simulink model "Complete_Model"  (R2023a)
settings: Solver=VariableStepAuto  StartTime=0.0  StopTime=22  MinStep=5e-5  RelTol=1e-3
values from neighbouring files (symbols this model uses):
  Cbus = 80e-3*n_wt_agg*u^2    (= 1.33333333333)    [param.m]
  D = 1e-3    (= 0.001)    [param.m]
  f = 50    [param.m]
  Fs = Vs * sqrt(2/3)/(2*pi*f)    [param.m]
  fsw = 4e3    (= 4000)    [param.m]
  Idr_rated = sqrt(2)*Is    [param.m]
  J = 127*n_wt_agg    (= 19050)    [param.m]
  ki_id = (wni^2)*Lr*sigma    (= 3.27733001332)    [param.m]
  ki_idg = (wnig^2)*Lg    (= 0.263189450696)    [param.m]
  ki_iq = ki_id    (= 3.27733001332)    [param.m]
  ki_iqg = ki_idg    (= 0.263189450696)    [param.m]
  ki_v = -300000*n_wt_agg    (= -45000000)    [param.m]
  Kopt = ((0.5*rho*pi*(Radius^5)*Cp_max)/(lambda_opt^3))    (= 296454.198186)    [param.m]
  kp_id = (2*wni*sigma*Lr) - Rr    (= 0.00384733333333)    [param.m]
  kp_idg = ((2*wnig*Lg)-Rg)    (= 0.00167538274858)    [param.m]
  kp_iq = kp_id    (= 0.00384733333333)    [param.m]
  kp_iqg = kp_idg    (= 0.00167538274858)    [param.m]
  kp_v = -1000*n_wt_agg    (= -150000)    [param.m]
  Kpg = 1/(1.5*Vr*sqrt(2/3))    [param.m]
  Kqg = -Kpg    [param.m]
  lambda_opt = 7.2    [param.m]
  Lg = 400e-6 /n_wt_agg    (= 2.66666666667e-06)    [param.m]
  Lm = 2.5e-3 /n_wt_agg    (= 1.66666666667e-05)    [param.m]
  Lr = Lm + Lsi    (= 1.72466666667e-05)    [param.m]
  Ls = Lm + Lsi    (= 1.72466666667e-05)    [param.m]
  Lsi = 0.087e-3 /n_wt_agg    (= 5.8e-07)    [param.m]
  N = 100    [param.m]
  n_wt_agg = 150    [param.m]
  p = 2    [param.m]
  Ps = 2e6*n_wt_agg    (= 300000000)    [param.m]
  Radius = 42    [param.m]
  Rg = 20e-6 /n_wt_agg    (= 1.33333333333e-07)    [param.m]
  rho = 1.225    [param.m]
  Rr = 2.9e-3 /n_wt_agg    (= 1.93333333333e-05)    [param.m]
  Rs = 2.6e-3 /n_wt_agg    (= 1.73333333333e-05)    [param.m]
  sigma = 1 - Lm^2 / (Ls*Lr)    (= 0.0661284179513)    [param.m]
  smax = 1/3    (= 0.333333333333)    [param.m]
  tab_lambda = [0.1:0.01:11.8]    [param.m]
  Vbus = 1150/u    (= 3450)    [param.m]
  Vs = 690    [param.m]
undefined symbols (not defined in the file or in its folder): Cp, D1, Delta, DiscIntNeverNeededParam, magnitude
libraries: spsSecondOrderFilterLib x133, spsThreePhaseVIMeasurementLib x58, spsPower3phInstantaneousLib x52, simulink x41, spsThreePhaseTransformerTwoWindingsLib x27, spsUniversalBridgeLib x22, spsPWMGenerator2LevelLib x22, spsSeriesRLCBranchLib x19, spsFirstOrderFilterLib x18, spsThreePhasePISectionLineLib x17, spsGroundLib x17, spsThreePhaseSeriesRLCBranchLib x17
(part 6 of 15)

== system Complete_Model/HVDC Link/Wind farm side control ==
  note: Active Power Control
  note: Reactive Power Control
  P_windfarm : Inport
  Pref_windfarm : Inport  Port=2
  Q_windfarm : Inport  Port=3
  Qref_windfarm : Inport  Port=4
  Clarke - Park Transform : eeTransforms/Park Transform  AxisAlignment=D-axis  PowerInvariant=off
      settings: LibrarySourceBlock=|
      | ee_sl_lib/Mathematical
      | Transforms/Park Transform
  Clarke - Park Transform1 : eeTransforms/Park Transform  AxisAlignment=D-axis  PowerInvariant=off
      settings: LibrarySourceBlock=|
      | ee_sl_lib/Mathematical
      | Transforms/Park Transform
  Constant : Constant  Value=0
  Constant1 : Constant  Value=0
  Demux{1..7} (7 alike) : Demux  Outputs=2
      Demux2 settings: DisplayOption=none
      Demux3 settings: DisplayOption=none
  Divide : Product  Inputs=*/
  Divide2 : Product  Inputs=*/
  First-Order Filter{1,3..11} (10 alike) : spsFirstOrderFilterLib/First-Order Filter  FilterType=Lowpass  Tc=0.015915  Ts=7.4074e-05  Initialize=on  Vac_Init=[0, 0, 50]  Vdc_Init=1.2247  PlotResponse=off  FreqRange="[0,  200,  1]"
      settings: AttributesFormatString=Tc:%<Tc>\nIC:%<Vdc_Init>
  First-Order Filter13 : spsFirstOrderFilterLib/First-Order Filter  FilterType=Lowpass  Tc=0.015915*2  Ts=7.4074e-05  Initialize=on  Vac_Init=[0, 0, 50]  Vdc_Init=1.2247  PlotResponse=off  FreqRange="[0,  200,  1]"
      settings: AttributesFormatString=Tc:%<Tc>\nIC:%<Vdc_Init>
  First-Order Filter2 : spsFirstOrderFilterLib/First-Order Filter  FilterType=Lowpass  Tc=0.015915*2  Ts=7.4074e-05  Initialize=on  Vac_Init=[0, 0, 50]  Vdc_Init=1.2247  PlotResponse=off  FreqRange="[0,  200,  1]"
      settings: AttributesFormatString=Tc:%<Tc>\nIC:%<Vdc_Init>
  Gain : Gain  Gain=0.1947+0.178
  Gain1 : Gain  Gain=0.1947+0.178
  Gain3 : Gain  Gain=-1
  Gain5 : Gain  Gain=-1
  I_max : Constant  Value=1.1
  Inverse Clarke - Park Transform : eeTransforms/Inverse Park Transform  AxisAlignment=D-axis  PowerInvariant=off
      settings: LibrarySourceBlock=|
      | ee_sl_lib/Mathematical
      | Transforms/Inverse
      | Park Transform
  Math Function3 : Math  Operator=magnitude^2  SignedPower=on
  Math Function4 : Math  Operator=magnitude^2  SignedPower=on
  Math Function5 : Math  Operator=sqrt  SignedPower=on
  Mux : Mux  Inputs=3
  Mux1 : Mux  Inputs=2
  PID Controller2 : slpidlib/PID Controller  Controller=PI  Form=Parallel  TimeDomain=Continuous-time  UseExternalTs=off  SampleTime=-1  IntegratorMethod=Forward Euler  FilterMethod=Forward Euler  ControllerParametersSource=internal  P=2  I=4  UseKiTs=off  D=0  N=100  UseFilter=on  TunerSelectOption=Transfer Function Based (PID Tuner App)  InitialConditionSource=internal  InitialConditionForIntegrator=0  InitialConditionForFilter=0  DifferentiatorICPrevScaledInput=0  ExternalReset=none  TrackingMode=off  Kt=1  LimitOutput=on  SatLimitsSource=internal  UpperSaturationLimit=1.1  LowerSaturationLimit=-1.1  LinearizeAsGain=on  AntiWindupMode=clamping  Kb=1  LimitIntegrator=on  UpperIntegratorSaturationLimit=20  LowerIntegratorSaturationLimit=-20  POutMin=[]  POutMax=[]  IOutMin=[]  IOutMax=[]  DOutMin=[]  DOutMax=[]  NOutMin=[]  NOutMax=[]  SumOutMin=[]  SumOutMax=[]  SaturationOutMin=[]  SaturationOutMax=[]  PParamMin=[]  PParamMax=[]  IParamMin=[]  IParamMax=[]  DParamMin=[]  DParamMax=[]  NParamMin=[]  NParamMax=[]  KbParamMin=[]  KbParamMax=[]  KtParamMin=[]  KtParamMax=[]  KbOutMin=[]  KbOutMax=[]  KtOutMin=[]  KtOutMax=[]  IntegratorOutMin=[]  IntegratorOutMax=[]  FilterOutMin=[]  FilterOutMax=[]  SumI1OutMin=[]  SumI1OutMax=[]  SumI2OutMin=[]  SumI2OutMax=[]  SumI3OutMin=[]  SumI3OutMax=[]  SumI4OutMin=[]  SumI4OutMax=[]  SumDOutMin=[]  SumDOutMax=[]  DifferentiatorOutMin=[]  DifferentiatorOutMax=[]  FilterDiffOutCoefMin=[]  FilterDiffOutCoefMax=[]  ReciprocalOutMin=[]  ReciprocalOutMax=[]  SumDenOutMin=[]  SumDenOutMax=[]  SumNumOutMin=[]  SumNumOutMax=[]  DivideOutMin=[]  DivideOutMax=[]  UdiffTsProdOutMin=[]  UdiffTsProdOutMax=[]  NTsProdOutMin=[]  NTsProdOutMax=[]  UintegralTsProdOutMin=[]  UintegralTsProdOutMax=[]  UngainTsProdOutMin=[]  UngainTsProdOutMax=[]  ClampingZeroOutDataTypeStr=Inherit: Inherit via back propagation  ClampingZeroOutMin=[]  ClampingZeroOutMax=[]  FilterDenConstantOutDataTypeStr=Inherit: Inherit via back propagation  FilterDenConstantOutMin=[]  FilterDenConstantOutMax=[]  PCopyOutDataTypeStr=Inherit: Inherit via back propagation  PCopyOutMin=[]  PCopyOutMax=[]  NCopyOutDataTypeStr=Inherit: Inherit via back propagation  NCopyOutMin=[]  NCopyOutMax=[]  IntegratorContinuousStateAttributes=''  IntegratorStateMustResolveToSignalObject=off  IntegratorRTWStateStorageClass=Auto  FilterContinuousStateAttributes=''  FilterStateMustResolveToSignalObject=off  FilterRTWStateStorageClass=Auto  ParallelPVariant=InternalParameters  IdealPVariant=Disabled  IVariant=InternalParameters  DVariant=Disabled  IntegratorVariant=Continuous  SatVariant=Enabled  AWVariant=ContClampingParallel  PCopyVariant=Disabled  TRVariant=Disabled  FdbkBlocksVariant=Forward  IdealPFdbkVariant=Disabled  SatFdbkVariant=Disabled  DerivativeFilterVariant=Disabled  NVariant=Disabled  NCopyVariant=Disabled_SignalSpec  FilterICVariant=Disabled  IntegratorICVariant=InternalICs  ExternalResetVariant=Disabled  TRSumVariant=Passthrough  SumFdbkVariant=Disabled  SumVariant=Sum_PI  TsampFilterVariant=InternalTs  TsampNgainVariant=Passthrough  TsampIntegralVariant=Disabled_SignalSpec  ExternalSaturationOutDataTypeStr=Inherit: Same as second input
  PID Controller5 : slpidlib/PID Controller  Controller=PI  Form=Parallel  TimeDomain=Continuous-time  UseExternalTs=off  SampleTime=-1  IntegratorMethod=Forward Euler  FilterMethod=Forward Euler  ControllerParametersSource=internal  P=2  I=10  UseKiTs=off  D=0  N=100  UseFilter=on  TunerSelectOption=Transfer Function Based (PID Tuner App)  InitialConditionSource=internal  InitialConditionForIntegrator=0  InitialConditionForFilter=0  DifferentiatorICPrevScaledInput=0  ExternalReset=none  TrackingMode=off  Kt=1  LimitOutput=on  SatLimitsSource=internal  UpperSaturationLimit=1.1  LowerSaturationLimit=-1.1  LinearizeAsGain=on  AntiWindupMode=clamping  Kb=1  LimitIntegrator=on  UpperIntegratorSaturationLimit=20  LowerIntegratorSaturationLimit=-20  POutMin=[]  POutMax=[]  IOutMin=[]  IOutMax=[]  DOutMin=[]  DOutMax=[]  NOutMin=[]  NOutMax=[]  SumOutMin=[]  SumOutMax=[]  SaturationOutMin=[]  SaturationOutMax=[]  PParamMin=[]  PParamMax=[]  IParamMin=[]  IParamMax=[]  DParamMin=[]  DParamMax=[]  NParamMin=[]  NParamMax=[]  KbParamMin=[]  KbParamMax=[]  KtParamMin=[]  KtParamMax=[]  KbOutMin=[]  KbOutMax=[]  KtOutMin=[]  KtOutMax=[]  IntegratorOutMin=[]  IntegratorOutMax=[]  FilterOutMin=[]  FilterOutMax=[]  SumI1OutMin=[]  SumI1OutMax=[]  SumI2OutMin=[]  SumI2OutMax=[]  SumI3OutMin=[]  SumI3OutMax=[]  SumI4OutMin=[]  SumI4OutMax=[]  SumDOutMin=[]  SumDOutMax=[]  DifferentiatorOutMin=[]  DifferentiatorOutMax=[]  FilterDiffOutCoefMin=[]  FilterDiffOutCoefMax=[]  ReciprocalOutMin=[]  ReciprocalOutMax=[]  SumDenOutMin=[]  SumDenOutMax=[]  SumNumOutMin=[]  SumNumOutMax=[]  DivideOutMin=[]  DivideOutMax=[]  UdiffTsProdOutMin=[]  UdiffTsProdOutMax=[]  NTsProdOutMin=[]  NTsProdOutMax=[]  UintegralTsProdOutMin=[]  UintegralTsProdOutMax=[]  UngainTsProdOutMin=[]  UngainTsProdOutMax=[]  ClampingZeroOutDataTypeStr=Inherit: Inherit via back propagation  ClampingZeroOutMin=[]  ClampingZeroOutMax=[]  FilterDenConstantOutDataTypeStr=Inherit: Inherit via back propagation  FilterDenConstantOutMin=[]  FilterDenConstantOutMax=[]  PCopyOutDataTypeStr=Inherit: Inherit via back propagation  PCopyOutMin=[]  PCopyOutMax=[]  NCopyOutDataTypeStr=Inherit: Inherit via back propagation  NCopyOutMin=[]  NCopyOutMax=[]  IntegratorContinuousStateAttributes=''  IntegratorStateMustResolveToSignalObject=off  IntegratorRTWStateStorageClass=Auto  FilterContinuousStateAttributes=''  FilterStateMustResolveToSignalObject=off  FilterRTWStateStorageClass=Auto  ParallelPVariant=InternalParameters  IdealPVariant=Disabled  IVariant=InternalParameters  DVariant=Disabled  IntegratorVariant=Continuous  SatVariant=Enabled  AWVariant=ContClampingParallel  PCopyVariant=Disabled  TRVariant=Disabled  FdbkBlocksVariant=Forward  IdealPFdbkVariant=Disabled  SatFdbkVariant=Disabled  DerivativeFilterVariant=Disabled  NVariant=Disabled  NCopyVariant=Disabled_SignalSpec  FilterICVariant=Disabled  IntegratorICVariant=InternalICs  ExternalResetVariant=Disabled  TRSumVariant=Passthrough  SumFdbkVariant=Disabled  SumVariant=Sum_PI  TsampFilterVariant=InternalTs  TsampNgainVariant=Passthrough  TsampIntegralVariant=Disabled_SignalSpec  ExternalSaturationOutDataTypeStr=Inherit: Same as second input
  PI_regulator 1 : Subsystem  mask(PI regulator with anti-windup - type 2){max_i=2, min_i=-2, Ki=6, i_ic=0, Kp=0.6, Kf=1.0, max_out=2, min_out=-2, Ts=7.4074e-05}
      mask prompts: max_i "Integrator maximum  limit (pu):"; min_i "Integrator minimum  limit (pu):"; Ki "Integrator gain (pu/s):"; i_ic "Integrator initial condition (pu):"; Kp "Proportional gain:"; Kf "Feadback gain :"; max_out "Output maximum limit (pu):"; min_out "Output minimum limit (pu):"; Ts "Sample time: (s)"
      mask help:
      | The error signal (input 1 - input 2) is integrated with dynamic limits (Integrator min and max limits).
      | A back-calculation anti-windup method discharges the integrator on block saturation (output min and max limits) using a feedback loop having a gain coefficient.
      mask Display: disp('PI \n(Type 2)')
  PI_regulator 2 : Subsystem  mask(PI regulator with anti-windup - type 2){max_i=2, min_i=-2, Ki=6, i_ic=0, Kp=0.6, Kf=1.0, max_out=2, min_out=-2, Ts=7.4074e-05}
      mask prompts: max_i "Integrator maximum  limit (pu):"; min_i "Integrator minimum  limit (pu):"; Ki "Integrator gain (pu/s):"; i_ic "Integrator initial condition (pu):"; Kp "Proportional gain:"; Kf "Feadback gain :"; max_out "Output maximum limit (pu):"; min_out "Output minimum limit (pu):"; Ts "Sample time: (s)"
      mask help:
      | The error signal (input 1 - input 2) is integrated with dynamic limits (Integrator min and max limits).
      | A back-calculation anti-windup method discharges the integrator on block saturation (output min and max limits) using a feedback loop having a gain coefficient.
      mask Display: disp('PI \n(Type 2)')
  Park Transform3 : eeTransforms/Park Transform  AxisAlignment=D-axis  PowerInvariant=on
      settings: LibrarySourceBlock=|
      | ee_sl_lib/Mathematical
      | Transforms/Park Transform
  Saturation : Saturate  UpperLimit=1.1  LowerLimit=-1.1
  Saturation Dynamic : simulink/Discontinuities/Saturation Dynamic  OutMin=[]  OutMax=[]  OutDataTypeStr=Inherit: Same as second input  OutDataType=fixdt(1, 16)  OutScaling=2^-10  DoSatur=off
  Saturation3 : Saturate  UpperLimit=1.1  LowerLimit=-1.1
  Scope12 : Scope
  Scope3 : Scope  NumInputPorts=2
  Selector1 : Selector  InputPortWidth=3  IndexOptions=Index vector (dialog)  Indices=[1 2]  OutputSizes=1
  Selector2 : Selector  InputPortWidth=3  IndexOptions=Index vector (dialog)  Indices=[1 2]  OutputSizes=1
  Selector3 : Selector  InputPortWidth=3  IndexOptions=Index vector (dialog)  Indices=[1 2]  OutputSizes=1
  Simple PLL : Trigonometry  Operator=atan2
  Simple PLL1 : Trigonometry  Operator=atan2
  Step : Step  Time=0.3  Before=1  After=-1  SampleTime=0
  Sum1 : Sum  Inputs=+|-|+  InputSameDT=on  SaturateOnIntegerOverflow=on  OutDataTypeStr=Inherit: Same as first input
  Sum2 : Sum  Inputs=-+  SaturateOnIntegerOverflow=on
  Sum3 : Sum  Inputs=--+  InputSameDT=on  SaturateOnIntegerOverflow=on  OutDataTypeStr=Inherit: Same as first input
  Sum4 : Sum  Inputs=+-|
  Sum5 : Sum  Inputs=++|
  Sum7 : Sum  Inputs=+-|
  Sum9 : Sum  Inputs=++|
  Switch : Switch  Criteria=u2 > Threshold  SaturateOnIntegerOverflow=off  InputSameDT=off
  To File : ToFile  Filename=Iabc_windfarm_hvdc  SaveFormat=Timeseries
  To Workspace : ToWorkspace  VariableName=Ewind_d  SaveFormat=Timeseries  SampleTime=-1
  To Workspace1 : ToWorkspace  VariableName=Ewind_q  SaveFormat=Timeseries  SampleTime=-1
  To Workspace2 : ToWorkspace  VariableName=Iwind_q  SaveFormat=Timeseries  SampleTime=-1
  To Workspace3 : ToWorkspace  VariableName=Iwind_d  SaveFormat=Timeseries  SampleTime=-1
  To Workspace4 : ToWorkspace  VariableName=Iwind_ref_d  SaveFormat=Timeseries  SampleTime=-1
  To Workspace5 : ToWorkspace  VariableName=Iwind_ref_q  SaveFormat=Timeseries  SampleTime=-1
  To Workspace6 : ToWorkspace  VariableName=Ewind_ref_d  SaveFormat=Timeseries  SampleTime=-1
  To Workspace7 : ToWorkspace  VariableName=Ewind_ref_q  SaveFormat=Timeseries  SampleTime=-1
  To Workspace8 : ToWorkspace  VariableName=Uconv_wind_d  SaveFormat=Timeseries  SampleTime=-1
  To Workspace9 : ToWorkspace  VariableName=Uconv_wind_q  SaveFormat=Timeseries  SampleTime=-1
  ToFile_Ewind_d : ToFile  Filename=Ewind_d.mat  SaveFormat=Timeseries
  ToFile_Ewind_q : ToFile  Filename=Ewind_q.mat  SaveFormat=Timeseries
  ToFile_Ewind_ref_d : ToFile  Filename=Ewind_ref_d.mat  SaveFormat=Timeseries
  ToFile_Ewind_ref_q : ToFile  Filename=Ewind_ref_q.mat  SaveFormat=Timeseries
  ToFile_Iwind_d : ToFile  Filename=Iwind_d.mat  SaveFormat=Timeseries
  ToFile_Iwind_q : ToFile  Filename=Iwind_q.mat  SaveFormat=Timeseries
  ToFile_Iwind_ref_d : ToFile  Filename=Iwind_ref_d.mat  SaveFormat=Timeseries
  ToFile_Iwind_ref_q : ToFile  Filename=Iwind_ref_q.mat  SaveFormat=Timeseries
  ToFile_Uconv_wind_d : ToFile  Filename=Uconv_wind_d.mat  SaveFormat=Timeseries
  ToFile_Uconv_wind_q : ToFile  Filename=Uconv_wind_q.mat  SaveFormat=Timeseries
  abc->alpha/beta Clarke : Subsystem
  abc->alpha/beta1 : Subsystem
  Vref_abc : Outport
  tag scope: Ewindfarm_dq=global, Idmes_hvdc_wf=global, Idref_hvdc_wf=global, Iqmes_hvdc_wf=global, Iqref_hvdc_wf=global, Iwindfarm_dq=global, Vdref_hvdc_wf=global, Vqref_hvdc_wf=global, theta_atan_windfarm=global
  wires:
  Constant/1 -> Mux/3
  Inverse Clarke - Park Transform/1 -> Vref_abc/1
  [Ewindfarm_d] -> Sum1/1
  Mux/1 -> Inverse Clarke - Park Transform/1
  Gain1/1 -> Sum3/2
  PI_regulator 2/1(Out) -> Sum3/1
  Demux3/1 -> Gain1/1, PI_regulator 1/1(Ord)  "I_ref_d"
  [Ewindfarm_q] -> Sum3/3
  Gain/1 -> Sum1/3
  PI_regulator 1/1(Out) -> Sum1/2
  Demux2/2 -> PI_regulator 2/2(Meas)  "Iv_q"
  Demux2/1 -> PI_regulator 1/2(Meas)  "Iv_d"
  Demux3/2 -> PI_regulator 2/1(Ord), Gain/1  "I_ref_q"
  Saturation/1 -> Math Function4/1, [Idref_hvdc_wf], Mux1/1, First-Order Filter7/1
  Math Function4/1 -> Sum2/1
  Sum2/1 -> Math Function5/1
  Math Function5/1 -> Gain3/1, Saturation Dynamic/1  "iqmax"
  Math Function3/1 -> Sum2/2
  Mux1/1 -> Demux3/1  "Idq_ref"
  I_max/1 -> Math Function3/1
  P_windfarm/1 -> Sum7/2
  Pref_windfarm/1 -> Divide/1, Sum7/1
  [Ewindfarm_d] -> Divide/2
  Divide/1 -> First-Order Filter2/1
  Saturation Dynamic/1 -> Mux1/2, First-Order Filter6/1, [Iqref_hvdc_wf]
  Switch/1 -> Saturation Dynamic/2
  Gain5/1 -> Switch/3
  Constant1/1 -> Switch/1
  Q_windfarm/1 -> Sum4/2
  Qref_windfarm/1 -> Divide2/1, Sum4/1
  Step/1 -> Switch/2
  Sum4/1 -> PID Controller2/1
  Gain3/1 -> Saturation Dynamic/3
  Demux5/2 -> [Ewindfarm_q], First-Order Filter4/1
  Demux5/1 -> [Ewindfarm_d], First-Order Filter5/1
  Demux4/1 -> Simple PLL/2
  Clarke - Park Transform/1 -> Selector2/1
  [Eabc_windfarm] -> Clarke - Park Transform/1, abc->alpha/beta Clarke/1(abc)
  Demux4/2 -> Simple PLL/1
  Simple PLL/1 -> [theta_atan_windfarm], Clarke - Park Transform/2  "theta_g"
  abc->alpha/beta Clarke/1(alpha/beta) -> Demux4/1
  [Iabc_windfarm] -> To File/1, Clarke - Park Transform1/1
  Selector2/1 -> [Ewindfarm_dq], Demux5/1  "E_dq"
  Clarke - Park Transform1/1 -> Selector1/1
  Demux1/2 -> First-Order Filter8/1, [Iqmes_hvdc_wf]
  Selector1/1 -> [Iwindfarm_dq], Demux2/1, Demux1/1  "I_dq"
  Demux1/1 -> First-Order Filter9/1, [Idmes_hvdc_wf]
  Sum5/1 -> Saturation/1  "idref"
  First-Order Filter2/1 -> Sum5/1
  Sum7/1 -> PID Controller5/1
  PID Controller5/1 -> Sum5/2
  First-Order Filter1/1 -> ToFile_Ewind_ref_d/1, To Workspace6/1  "Ewind_ref_d"
  First-Order Filter3/1 -> To Workspace7/1, ToFile_Ewind_ref_q/1  "Ewind_ref_q"
  First-Order Filter4/1 -> To Workspace1/1, ToFile_Ewind_q/1  "Ewind_q"
  First-Order Filter5/1 -> To Workspace/1, ToFile_Ewind_d/1  "Ewind_d"
  First-Order Filter6/1 -> ToFile_Iwind_ref_q/1, To Workspace5/1
  First-Order Filter7/1 -> To Workspace4/1, ToFile_Iwind_ref_d/1
  First-Order Filter8/1 -> To Workspace2/1, ToFile_Iwind_q/1  "Iwind_q"
  First-Order Filter9/1 -> ToFile_Iwind_d/1, To Workspace3/1
  abc->alpha/beta1/1(alpha/beta) -> Scope12/1, Demux6/1
  Selector3/1 -> Demux7/1  "E_dq"
  Demux6/1 -> Simple PLL1/2
  Demux7/1 -> First-Order Filter11/1, Scope3/1
  Demux7/2 -> First-Order Filter10/1, Scope3/2
  Demux6/2 -> Simple PLL1/1
  First-Order Filter11/1 -> To Workspace8/1, ToFile_Uconv_wind_d/1  "Uconv_wind_d"
  Simple PLL1/1 -> Park Transform3/2  "theta_g"
  [Uconv_windfarm] -> Park Transform3/1, abc->alpha/beta1/1(abc)
  Park Transform3/1 -> Selector3/1
  First-Order Filter10/1 -> To Workspace9/1, ToFile_Uconv_wind_q/1  "Uconv_wind_q"
  Saturation3/1 -> Gain5/1
  Sum9/1 -> Saturation3/1  "iqref"
  PID Controller2/1 -> Sum9/2
  Divide2/1 -> First-Order Filter13/1
  First-Order Filter13/1 -> Sum9/1
  [Ewindfarm_d] -> Divide2/2
  Sum1/1 -> [Vdref_hvdc_wf], Mux/1, First-Order Filter1/1
  Sum3/1 -> Mux/2, [Vqref_hvdc_wf], First-Order Filter3/1
  [theta_atan_windfarm] -> Inverse Clarke - Park Transform/2
  [theta_atan_windfarm] -> Clarke - Park Transform1/2

== system Complete_Model/HVDC Link/big scope ==
  HVDC BE Side : Scope  NumInputPorts=9
  HVDC Windfarm Side : Scope  NumInputPorts=9
  Mux{1..8,13} (9 alike) : Mux  Inputs=2
  wires:
  [Iqmes_hvdc_wf] -> Mux1/2  "iq"
  Mux1/1 -> HVDC Windfarm Side/2
  Mux3/1 -> HVDC Windfarm Side/5
  [Idref_hvdc_wf] -> Mux3/1  "id_ref"
  [Vqref_hvdc_wf] -> HVDC Windfarm Side/6  "vqr_ref"
  [Eabc_windfarm] -> HVDC Windfarm Side/7  "Eabc"
  [Iabc_windfarm] -> HVDC Windfarm Side/8  "Iabc"
  [I_DC] -> HVDC Windfarm Side/9  "Idc"
  [Iabc_grid] -> HVDC BE Side/8  "Iabc"
  [Idref_hvdc_BE] -> Mux7/1  "id_ref"
  [Eabc_grid] -> HVDC BE Side/7  "Eabc"
  [Idmes_hvdc_BE] -> Mux7/2  "id_mes"
  [Vdc_ref] -> Mux4/1  "Vdc_ref"
  Mux7/1 -> HVDC BE Side/5
  [Vdcp_mes] -> Mux4/2  "Vdc_mes"
  Mux5/1 -> HVDC BE Side/2
  [Iqmes_hvdc_BE] -> Mux5/2  "iq_mes"
  Mux4/1 -> HVDC BE Side/1
  [Iqref_hvdc_BE] -> Mux5/1  "iq_ref"
  [Qref_hvdc_BE] -> Mux13/1  "Q_ref"
  [Qmes_hvdc_BE] -> Mux13/2  "Q_mes"
  Mux13/1 -> HVDC BE Side/4
  [Iqref_hvdc_wf] -> Mux1/1  "iq_ref"
  [Vdref_hvdc_wf] -> HVDC Windfarm Side/3  "vdr_ref"
  [Idmes_hvdc_wf] -> Mux3/2  "id"
  [Pref_hvdc_wfside] -> Mux2/1  "Pref"
  [P_hvdc_wfside] -> Mux2/2  "P"
  Mux2/1 -> HVDC Windfarm Side/1
  [Qref_hvdc_wfside] -> Mux6/1  "Qref"
  [Q_hvdc_wfside] -> Mux6/2  "Q"
  Mux6/1 -> HVDC Windfarm Side/4
  Mux8/1 -> HVDC BE Side/9
  [P_hvdc_BE] -> Mux8/2  "P_BE"
  [P_hvdc_wfside] -> Mux8/1  "P_wfside"

== system Complete_Model/Equivalent Wind Park/300MW Eq WT1/Aerodynamic Model ==
  note: v_{wind}
  note: C_p (\lambda)
  note: \lambda = \Omega_m R / v_{wind}
  note: Cp
  note: \Omega_m blades
  note: Motor convention : Generated power
      |  is negative
  note: Gearbox ratio
  Vw : Inport
  C_p (\lambda) : Lookup_n-D  NumberOfTableDimensions=1  BreakpointsForDimension1=tab_lambda  Table=Cp  InternalRulePriority=Speed  RndMeth=Simplest
  Constant : Constant  Value=Radius
  Divide : Product  Inputs=*/
  Divide1 : Product  Inputs=**/
  Fcn2 : Fcn  Expr=0.5*rho*pi*Radius^2*u(2)^3*u(1)
  Gain : Gain  Gain=1/N
  Gain2 : Gain  Gain=n_wt_agg
  Gain4 : Gain  Gain=-1
  Mux2 : Mux  Inputs=2
  Tm : Outport
  tag scope: P_m=global, Tm=global, lambda=global
  wires:
  [omega_m] -> Gain/1
  Gain/1 -> Divide1/2
  Vw/1 -> Divide1/3, Mux2/2
  Divide1/1 -> C_p (\lambda)/1, [lambda]  "lambda"
  C_p (\lambda)/1 -> Mux2/1
  Mux2/1 -> Fcn2/1
  Fcn2/1 -> Gain2/1
  Gain2/1 -> [P_m], Gain4/1
  [omega_m] -> Divide/2
  Divide/1 -> [Tm], Tm/1
  Constant/1 -> Divide1/1
  Gain4/1 -> Divide/1

== system Complete_Model/Equivalent Wind Park/300MW Eq WT1/Grid Side Control ==
  Vbus_ref : Inport
  Qg_ref : Inport  Port=2
  Vbus : Inport  Port=3
  Ig_mes : Inport  Port=4
  Vs_mes : Inport  Port=5
  Demux : Demux  Outputs=2
  Demux1 : Demux  Outputs=2
  Gain : Gain  Gain=Kqg
  Gain1 : Gain  Gain=Kpg
  Normalized for PWM : Gain  Gain=1/(Vbus/2)
  "PI " : Subsystem
  PI id : Subsystem
  PI iq : Subsystem
  Sum : Sum  Inputs=|++
  Vs_mes1 : InportShadow  Port=5
  abc->alpha/beta : Subsystem
  abc->alpha/beta1 : Subsystem
  alpha/beta->abc : Subsystem
  alpha/beta->d/q : Subsystem
  alpha/beta->d/q1 : Subsystem
  angle2 : Subsystem
  cancellation of coupling terms : Subsystem
  d/q->alpha/beta : Subsystem
  Vabc_ref : Outport
  tag scope: Qg_ref=global, Vbus_ref=global, idg=global, idg_ref=global, iqg=global, iqg_ref=global, vdg=global, vqg=global
  wires:
  d/q->alpha/beta/1(D/Q) -> alpha/beta->abc/1(D/Q)
  Ig_mes/1 -> abc->alpha/beta/1(abc)
  abc->alpha/beta/1(alpha/beta) -> alpha/beta->d/q/1(D/Q)
  Normalized for PWM/1 -> Vabc_ref/1
  Vs_mes/1 -> angle2/1(Vs_mes)
  PI id/1(out) -> cancellation of coupling terms/1(Vd*)
  PI iq/1(out) -> cancellation of coupling terms/2(Vq*)
  alpha/beta->d/q/1(d/q) -> Demux/1
  Vbus_ref/1 -> [Vbus_ref], "PI "/1(ref)
  Gain/1 -> PI iq/1(ref), [iqg_ref]  "iqg"
  "PI "/1(out) -> Gain1/1  "Pg_ref"
  Gain1/1 -> [idg_ref], PI id/1(ref)  "idg"
  Qg_ref/1 -> [Qg_ref], Gain/1
  alpha/beta->abc/1(abc) -> Sum/1
  Sum/1 -> Normalized for PWM/1
  cancellation of coupling terms/1(out) -> d/q->alpha/beta/1(d/q)
  [Vg] -> abc->alpha/beta1/1(abc)
  abc->alpha/beta1/1(alpha/beta) -> alpha/beta->d/q1/1(D/Q)
  alpha/beta->d/q1/1(d/q) -> Demux1/1
  Demux1/2 -> [vqg]
  Demux1/1 -> [vdg]
  Vbus/1 -> "PI "/2(meas)
  Demux/1 -> [idg], PI id/2(meas)
  Demux/2 -> [iqg], PI iq/2(meas)
  [theta_grid] -> alpha/beta->d/q/2(theta)
  [theta_grid] -> d/q->alpha/beta/2(theta)
  [theta_grid] -> alpha/beta->d/q1/2(theta)
  angle2/1(theta_g) -> [theta_grid]
  Vs_mes1/1 -> Sum/2

== system Complete_Model/Equivalent Wind Park/300MW Eq WT1/Power Calculations ==
  Demux{,1..3} (4 alike) : Demux  Outputs=2
  Divide : Product  Inputs=**
  Gain : Gain  Gain=3/2
  Gain1 : Gain  Gain=-1
  Gain2 : Gain  Gain=-1
  Power (3ph, Instantaneous){1..4} (4 alike) : spsPower3phInstantaneousLib/Power (3ph, Instantaneous)
  Scope{1..8,10..13} (12 alike) : Scope
  Scope9 : Scope  NumInputPorts=2
  "Second-Order " : spsSecondOrderFilterLib/Second-Order Filter  FilterType=Lowpass  Fo=17  Zeta=0.7  Ts=7.4074e-05  Initialize=off  Vac_Init="[0,  0,  60]"  Vdc_Init=0  PlotResponse=off  FreqRange=[0, 500, 1]
      settings: AttributesFormatString=f0=%<Fo>
  Second-Order 1 : spsSecondOrderFilterLib/Second-Order Filter  FilterType=Lowpass  Fo=17  Zeta=0.7  Ts=7.4074e-05  Initialize=off  Vac_Init="[0,  0,  60]"  Vdc_Init=0  PlotResponse=off  FreqRange=[0, 500, 1]
      settings: AttributesFormatString=f0=%<Fo>
  Second-Order Low-Pass Filter {1..7} (7 alike) : spsSecondOrderFilterLib/Second-Order Filter  FilterType=Lowpass  Fo=17  Zeta=0.7  Ts=7.4074e-05  Initialize=off  Vac_Init="[0,  0,  60]"  Vdc_Init=0  PlotResponse=off  FreqRange=[0, 500, 1]
      settings: AttributesFormatString=f0=%<Fo>
  Subsystem : Subsystem
  Subsystem1 : Subsystem
  Subsystem2 : Subsystem
  Subsystem3 : Subsystem
  To Workspace1 : ToWorkspace  VariableName=Iout_d  SaveFormat=Timeseries  SampleTime=-1
  To Workspace2 : ToWorkspace  VariableName=Ig_d  SaveFormat=Timeseries  SampleTime=-1
  To Workspace21 : ToWorkspace  VariableName=Vout_d  SaveFormat=Timeseries  SampleTime=-1
  To Workspace3 : ToWorkspace  VariableName=Vg_d  SaveFormat=Timeseries  SampleTime=-1
  alpha/beta->d/q : Subsystem
  alpha/beta->d/q1 : Subsystem
  alpha/beta->d/q2 : Subsystem
  alpha/beta->d/q3 : Subsystem
  angle1 : Subsystem
  angle2 : Subsystem
  tag scope: Pg_mes=global, Pout=global, Pr_mes_machine=global, Ps_mes_machine=global, Qg_mes=global, Qout=global, Qr_mes_machine=global, Qs_mes_machine=global
  wires:
  "Power (3ph, Instantaneous)2"/2 -> Second-Order Low-Pass Filter 1/1
  Second-Order Low-Pass Filter 1/1 -> [Qout]  "Qout"
  Second-Order Low-Pass Filter 3/1 -> [Ps_mes_machine]  "P_stator"
  "Power (3ph, Instantaneous)1"/2 -> Second-Order Low-Pass Filter 6/1
  [Iout] -> Scope3/1, "Power (3ph, Instantaneous)2"/2
  "Power (3ph, Instantaneous)4"/2 -> Second-Order Low-Pass Filter 4/1
  [Ig] -> "Power (3ph, Instantaneous)1"/2, Scope1/1
  "Power (3ph, Instantaneous)3"/1 -> Second-Order Low-Pass Filter 3/1  "Ps"
  Second-Order Low-Pass Filter 4/1 -> [Qr_mes_machine]  "Q_rotor"
  Second-Order Low-Pass Filter 6/1 -> [Qg_mes]  "Q_rotor"
  "Second-Order "/1 -> Gain1/1, [Pout]  "Pout"
  [Ir_mes] -> Scope5/1, "Power (3ph, Instantaneous)4"/2
  Second-Order Low-Pass Filter 7/1 -> [Pg_mes]  "P_rotor"
  [Is_mes_machine] -> Scope4/1, "Power (3ph, Instantaneous)3"/2
  Second-Order Low-Pass Filter 5/1 -> [Pr_mes_machine]  "P_rotor"
  "Power (3ph, Instantaneous)2"/1 -> "Second-Order "/1
  [Vs_mes_machine] -> "Power (3ph, Instantaneous)3"/1
  Second-Order Low-Pass Filter 2/1 -> [Qs_mes_machine]  "Q_stator"
  [Vg] -> "Power (3ph, Instantaneous)1"/1
  "Power (3ph, Instantaneous)3"/2 -> Second-Order Low-Pass Filter 2/1
  [Vout] -> "Power (3ph, Instantaneous)2"/1
  "Power (3ph, Instantaneous)4"/1 -> Second-Order Low-Pass Filter 5/1  "Pr"
  "Power (3ph, Instantaneous)1"/1 -> Second-Order Low-Pass Filter 7/1  "Pr"
  [Vr_mes] -> "Power (3ph, Instantaneous)4"/1
  [Vout] -> angle2/1(Vs_mes), Subsystem/1(abc)
  Subsystem/1(alpha/beta) -> alpha/beta->d/q/1(D/Q)
  alpha/beta->d/q/1(d/q) -> Demux/1
  Demux/1 -> Divide/1, To Workspace21/1, Scope2/1
  Demux/2 -> Scope6/1
  Demux1/2 -> Scope8/1
  Demux1/1 -> Divide/2, Scope7/1, To Workspace1/1
  alpha/beta->d/q1/1(d/q) -> Demux1/1
  Subsystem1/1(alpha/beta) -> alpha/beta->d/q1/1(D/Q)
  angle2/1(theta_g) -> alpha/beta->d/q1/2(theta), alpha/beta->d/q/2(theta)
  [Iout] -> Subsystem1/1(abc)
  Divide/1 -> Gain/1
  Second-Order 1/1 -> Scope9/1  "Pout"
  Gain1/1 -> Scope9/2
  Gain/1 -> Gain2/1
  Gain2/1 -> Second-Order 1/1
  [Ig] -> Subsystem3/1(abc)
  angle1/1(theta_g) -> alpha/beta->d/q2/2(theta), alpha/beta->d/q3/2(theta)
  Subsystem3/1(alpha/beta) -> alpha/beta->d/q3/1(D/Q)
  alpha/beta->d/q3/1(d/q) -> Demux3/1
  Demux3/1 -> To Workspace2/1, Scope12/1
  Demux3/2 -> Scope13/1
  Demux2/2 -> Scope11/1
  Demux2/1 -> Scope10/1, To Workspace3/1
  alpha/beta->d/q2/1(d/q) -> Demux2/1
  Subsystem2/1(alpha/beta) -> alpha/beta->d/q2/1(D/Q)
  [Vg] -> Subsystem2/1(abc), angle1/1(Vs_mes)

== system Complete_Model/Equivalent Wind Park/300MW Eq WT1/Rotor Side Control ==
  note: Stator referred
  idr_ref : Inport
  Ir_mes : Inport  Port=2
  Vs_mes : Inport  Port=3
  theta_m_mes : Inport  Port=4
  speed_mes : Inport  Port=5
  D/Q->abc : Subsystem
  D/Q->d/q : Subsystem
  Demux : Demux  Outputs=2
  Gain : Gain  Gain=1/(-1.5*p*(Lm/Ls)*Fs)
  Gain1 : Gain  Gain=1/u
  Gain2 : Gain  Gain=1/u
  MPPT : Subsystem
  Normalized for PWM : Gain  Gain=1/(Vbus/2)
  PI id : Subsystem
  PI iq : Subsystem
  abc->alpha/beta : Subsystem
  angle : Subsystem
  cancellation of coupling terms : Subsystem
  d/q->D/Q : Subsystem
  Vabc_ref : Outport
  tag scope: Tr_ref=global, idr=global, idr_ref=global, iqr=global, iqr_ref=global, vrabc_ref=global
  wires:
  Ir_mes/1 -> Gain1/1
  theta_m_mes/1 -> angle/2(theta_m)
  Vs_mes/1 -> angle/1(Vs_mes)
  angle/1(theta_r) -> d/q->D/Q/2(theta), D/Q->d/q/2(theta)
  PI id/1(out) -> cancellation of coupling terms/1(Vd*)
  PI iq/1(out) -> cancellation of coupling terms/2(Vq*)
  cancellation of coupling terms/1(out) -> d/q->D/Q/1(d/q)  "Vdr_ref,Vqr_ref"
  speed_mes/1 -> cancellation of coupling terms/3(omega_m), MPPT/1(omega_m)
  D/Q->d/q/1(d/q) -> Demux/1  "i_dq = rotor ref frame"
  Demux/1 -> PI id/2(meas), [idr]
  Demux/2 -> [iqr], PI iq/2(meas)
  Gain/1 -> [iqr_ref], PI iq/1(ref)
  idr_ref/1 -> [idr_ref], PI id/1(ref)
  Gain1/1 -> abc->alpha/beta/1(abc)
  Normalized for PWM/1 -> Vabc_ref/1
  D/Q->abc/1(abc) -> Gain2/1
  Gain2/1 -> Normalized for PWM/1, [vrabc_ref]  "Rotor referred"
  abc->alpha/beta/1(alpha/beta) -> D/Q->d/q/1(D/Q)
  d/q->D/Q/1(D/Q) -> D/Q->abc/1(D/Q)
  MPPT/1(Tm,opt) -> [Tr_ref], Gain/1

== system Complete_Model/Equivalent Wind Park/300MW Eq WT1/Subsystem ==
  In1 : Inport
  Bus Selector : BusSelector  OutputSignals=Mechanical.Rotor angle thetam (rad),Mechanical.Rotor speed (wm),Mechanical.Electromagnetic torque Te (N*m)
  Second-Order Low-Pass Filter 3 : spsSecondOrderFilterLib/Second-Order Filter  FilterType=Lowpass  Fo=17  Zeta=0.7  Ts=7.4074e-05  Initialize=off  Vac_Init="[0,  0,  60]"  Vdc_Init=0  PlotResponse=off  FreqRange=[0, 500, 1]
      settings: AttributesFormatString=f0=%<Fo>
  tag scope: Tr=global, omega_m=global, theta=global
  wires:
  Bus Selector/2 -> Second-Order Low-Pass Filter 3/1  "<Rotor speed (wm)>"
  In1/1 -> Bus Selector/1
  Bus Selector/1 -> [theta]  "<Rotor angle thetam (rad)>"
  Bus Selector/3 -> [Tr]  "<Electromagnetic torque Te (N*m)>"
  Second-Order Low-Pass Filter 3/1 -> [omega_m]

== system Complete_Model/Equivalent Wind Park/300MW Eq WT1/Subsystem1 ==
  Constant4 : Constant  Value=0
  Step : Step  Time=50  After=-0.2*Ps  SampleTime=0
  Sum14 : Sum  Inputs=++|
  Out1 : Outport
  wires:
  Constant4/1 -> Sum14/2
  Step/1 -> Sum14/1
  Sum14/1 -> Out1/1

== system Complete_Model/Equivalent Wind Park/300MW Eq WT1/Subsystem2 ==
  Constant1 : Constant  Value=9
  Ramp1 : simulink/Sources/Ramp  slope=-0.4  start=13  InitialOutput=0
  Ramp2 : simulink/Sources/Ramp  slope=0.4  start=8  InitialOutput=0
  Sum1 : Sum  Inputs=++|
  Sum2 : Sum  Inputs=++|
  v_wind : Outport
  tag scope: v_wind=global
  wires:
  Sum2/1 -> [v_wind], v_wind/1
  Ramp1/1 -> Sum1/2
  Constant1/1 -> Sum2/2
  Sum1/1 -> Sum2/1
  Ramp2/1 -> Sum1/1

== system Complete_Model/Equivalent Wind Park/300MW Eq WT1/Subsystem8 ==
  Constant4 : Constant  Value=0
  Step : Step  Time=55  After=Idr_rated*0.2  SampleTime=0
  Sum14 : Sum  Inputs=++|
  Out1 : Outport
  wires:
  Step/1 -> Sum14/1
  Constant4/1 -> Sum14/2
  Sum14/1 -> Out1/1

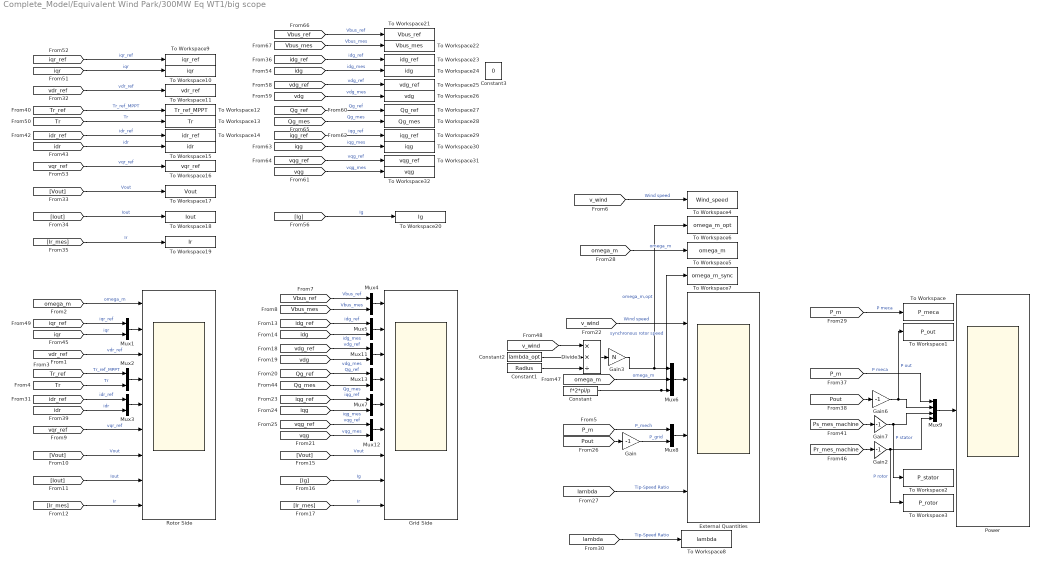
== system Complete_Model/Equivalent Wind Park/300MW Eq WT1/big scope ==
  Constant : Constant  Value=f*2*pi/p
  Constant1 : Constant  Value=Radius
  Constant2 : Constant  Value=lambda_opt
  Constant3 : Constant  Value=0
  Divide3 : Product  Inputs=**/
  External Quantities : Scope  NumInputPorts=4
  Gain{,2,6..7} (4 alike) : Gain  Gain=-1
  Gain3 : Gain  Gain=N
  Grid Side : Scope  NumInputPorts=9
  Mux{1..5,7..8,11..13} (10 alike) : Mux  Inputs=2
  Mux6 : Mux  Inputs=3
  Mux9 : Mux
  Power : Scope
  Rotor Side : Scope  NumInputPorts=9
  To Workspace : ToWorkspace  VariableName=P_meca  SaveFormat=Timeseries  SampleTime=-1
  To Workspace1 : ToWorkspace  VariableName=P_out  SaveFormat=Timeseries  SampleTime=-1
  To Workspace10 : ToWorkspace  VariableName=iqr  SaveFormat=Timeseries  SampleTime=-1
  To Workspace11 : ToWorkspace  VariableName=vdr_ref  SaveFormat=Timeseries  SampleTime=-1
  To Workspace12 : ToWorkspace  VariableName=Tr_ref_MPPT  SaveFormat=Timeseries  SampleTime=-1
  To Workspace13 : ToWorkspace  VariableName=Tr  SaveFormat=Timeseries  SampleTime=-1
  To Workspace14 : ToWorkspace  VariableName=idr_ref  SaveFormat=Timeseries  SampleTime=-1
  To Workspace15 : ToWorkspace  VariableName=idr  SaveFormat=Timeseries  SampleTime=-1
  To Workspace16 : ToWorkspace  VariableName=vqr_ref  SaveFormat=Timeseries  SampleTime=-1
  To Workspace17 : ToWorkspace  VariableName=Vout  SaveFormat=Timeseries  SampleTime=-1
  To Workspace18 : ToWorkspace  VariableName=Iout  SaveFormat=Timeseries  SampleTime=-1
  To Workspace19 : ToWorkspace  VariableName=Ir  SaveFormat=Timeseries  SampleTime=-1
  To Workspace2 : ToWorkspace  VariableName=P_stator  SaveFormat=Timeseries  SampleTime=-1
  To Workspace20 : ToWorkspace  VariableName=Ig  SaveFormat=Timeseries  SampleTime=-1
  To Workspace21 : ToWorkspace  VariableName=Vbus_ref  SaveFormat=Timeseries  SampleTime=-1
  To Workspace22 : ToWorkspace  VariableName=Vbus_mes  SaveFormat=Timeseries  SampleTime=-1
  To Workspace23 : ToWorkspace  VariableName=idg_ref  SaveFormat=Timeseries  SampleTime=-1
  To Workspace24 : ToWorkspace  VariableName=idg  SaveFormat=Timeseries  SampleTime=-1
  To Workspace25 : ToWorkspace  VariableName=vdg_ref  SaveFormat=Timeseries  SampleTime=-1
  To Workspace26 : ToWorkspace  VariableName=vdg  SaveFormat=Timeseries  SampleTime=-1
  To Workspace27 : ToWorkspace  VariableName=Qg_ref  SaveFormat=Timeseries  SampleTime=-1
  To Workspace28 : ToWorkspace  VariableName=Qg_mes  SaveFormat=Timeseries  SampleTime=-1
  To Workspace29 : ToWorkspace  VariableName=iqg_ref  SaveFormat=Timeseries  SampleTime=-1
  To Workspace3 : ToWorkspace  VariableName=P_rotor  SaveFormat=Timeseries  SampleTime=-1
  To Workspace30 : ToWorkspace  VariableName=iqg  SaveFormat=Timeseries  SampleTime=-1
  To Workspace31 : ToWorkspace  VariableName=vqg_ref  SaveFormat=Timeseries  SampleTime=-1
  To Workspace32 : ToWorkspace  VariableName=vqg  SaveFormat=Timeseries  SampleTime=-1
  To Workspace4 : ToWorkspace  VariableName=Wind_speed  SaveFormat=Timeseries  SampleTime=-1
  To Workspace5 : ToWorkspace  VariableName=omega_m  SaveFormat=Timeseries  SampleTime=-1
  To Workspace6 : ToWorkspace  VariableName=omega_m_opt  SaveFormat=Timeseries  SampleTime=-1
  To Workspace7 : ToWorkspace  VariableName=omega_m_sync  SaveFormat=Timeseries  SampleTime=-1
  To Workspace8 : ToWorkspace  VariableName=lambda  SaveFormat=Timeseries  SampleTime=-1
  To Workspace9 : ToWorkspace  VariableName=iqr_ref  SaveFormat=Timeseries  SampleTime=-1
  wires:
  [iqr] -> Mux1/2  "iqr"
  Mux1/1 -> Rotor Side/2
  [Tr_ref] -> Mux2/1  "Tr_ref_MPPT"
  Mux2/1 -> Rotor Side/4
  Mux3/1 -> Rotor Side/5
  [idr_ref] -> Mux3/1  "idr_ref"
  [vqr_ref] -> Rotor Side/6  "vqr_ref"
  [Vout] -> Rotor Side/7  "Vout"
  [Iout] -> Rotor Side/8  "Iout"
  [Ir_mes] -> Rotor Side/9  "Ir"
  [Ig] -> Grid Side/8  "Ig"
  [iqg_ref] -> Mux7/1  "iqg_ref"
  [Vout] -> Grid Side/7  "Vout"
  [Ir_mes] -> Grid Side/9  "Ir"
  [iqg] -> Mux7/2  "iqg_mes"
  [Vbus_ref] -> Mux4/1  "Vbus_ref"
  Mux7/1 -> Grid Side/5
  [Vbus_mes] -> Mux4/2  "Vbus_mes"
  Mux5/1 -> Grid Side/2
  [idg] -> Mux5/2  "idg_mes"
  Mux4/1 -> Grid Side/1
  [idg_ref] -> Mux5/1  "idg_ref"
  [omega_m] -> Rotor Side/1  "omega_m"
  Gain/1 -> Mux8/2  "P_grid"
  [Pout] -> Gain/1
  Mux8/1 -> External Quantities/3
  Mux6/1 -> External Quantities/2
  [v_wind] -> External Quantities/1  "Wind speed"
  [omega_m] -> Mux6/2  "omega_m"
  Constant/1 -> To Workspace7/1, Mux6/3  "synchronous rotor speed"
  [P_m] -> Mux8/1  "P_mech"
  [vdg_ref] -> Mux11/1  "vdg_ref"
  [vdg] -> Mux11/2  "vdg_mes"
  Mux11/1 -> Grid Side/3
  Gain6/1 -> To Workspace1/1, Mux9/2  "P out"
  [Ps_mes_machine] -> Gain7/1
  Gain7/1 -> To Workspace2/1, Mux9/3  "P stator"
  [Pr_mes_machine] -> Gain2/1
  [P_m] -> Mux9/1  "P meca"
  Mux9/1 -> Power/1
  [Pout] -> Gain6/1
  Gain2/1 -> To Workspace3/1, Mux9/4  "P rotor"
  [vqg] -> Mux12/2  "vqg_mes"
  [vqg_ref] -> Mux12/1  "vqg_ref"
  Mux12/1 -> Grid Side/6
  [Qg_ref] -> Mux13/1  "Qg_ref"
  [Qg_mes] -> Mux13/2  "Qg_mes"
  Mux13/1 -> Grid Side/4
  [iqr_ref] -> Mux1/1  "iqr_ref"
  [vdr_ref] -> Rotor Side/3  "vdr_ref"
  [Tr] -> Mux2/2  "Tr"
  [idr] -> Mux3/2  "idr"
  [v_wind] -> Divide3/1
  Constant2/1 -> Divide3/2
  Constant1/1 -> Divide3/3
  Divide3/1 -> Gain3/1
  Gain3/1 -> To Workspace6/1, Mux6/1  "omega_m,opt"
  [lambda] -> External Quantities/4  "Tip-Speed Ratio"
  [v_wind] -> To Workspace4/1  "Wind speed"
  [omega_m] -> To Workspace5/1  "omega_m"
  [P_m] -> To Workspace/1  "P meca"
  [lambda] -> To Workspace8/1  "Tip-Speed Ratio"
  [iqr_ref] -> To Workspace9/1  "iqr_ref"
  [iqr] -> To Workspace10/1  "iqr"
  [vdr_ref] -> To Workspace11/1  "vdr_ref"
  [Tr_ref] -> To Workspace12/1  "Tr_ref_MPPT"
  [Tr] -> To Workspace13/1  "Tr"
  [idr_ref] -> To Workspace14/1  "idr_ref"
  [idr] -> To Workspace15/1  "idr"
  [vqr_ref] -> To Workspace16/1  "vqr_ref"
  [Vout] -> To Workspace17/1  "Vout"
  [Iout] -> To Workspace18/1  "Iout"
  [Ir_mes] -> To Workspace19/1  "Ir"
  [Ig] -> To Workspace20/1  "Ig"
  [Vbus_ref] -> To Workspace21/1  "Vbus_ref"
  [Vbus_mes] -> To Workspace22/1  "Vbus_mes"
  [idg_ref] -> To Workspace23/1  "idg_ref"
  [idg] -> To Workspace24/1  "idg_mes"
  [vdg_ref] -> To Workspace25/1  "vdg_ref"
  [Qg_ref] -> To Workspace27/1  "Qg_ref"
  [vdg] -> To Workspace26/1  "vdg_mes"
  [Qg_mes] -> To Workspace28/1  "Qg_mes"
  [iqg_ref] -> To Workspace29/1  "iqg_ref"
  [iqg] -> To Workspace30/1  "iqg_mes"
  [vqg_ref] -> To Workspace31/1  "vqg_ref"
  [vqg] -> To Workspace32/1  "vqg_mes"

== system Complete_Model/Equivalent Wind Park/300MW Eq WT10/Aerodynamic Model ==
  note: Gearbox ratio
  note: Motor convention : Generated power
      |  is negative
  note: \Omega_m blades
  note: Cp
  note: \lambda = \Omega_m R / v_{wind}
  note: C_p (\lambda)
  note: v_{wind}
  Vw : Inport
  C_p (\lambda) : Lookup_n-D  NumberOfTableDimensions=1  BreakpointsForDimension1=tab_lambda  Table=Cp  InternalRulePriority=Speed  RndMeth=Simplest
  Constant : Constant  Value=Radius
  Divide : Product  Inputs=*/
  Divide1 : Product  Inputs=**/
  Fcn2 : Fcn  Expr=0.5*rho*pi*Radius^2*u(2)^3*u(1)
  Gain : Gain  Gain=1/N
  Gain2 : Gain  Gain=n_wt_agg
  Gain4 : Gain  Gain=-1
  Mux2 : Mux  Inputs=2
  Tm : Outport
  tag scope: P_m3=global, Tm3=global, lambda3=global
  wires:
  Gain4/1 -> Divide/1
  Constant/1 -> Divide1/1
  Divide/1 -> Tm/1, [Tm3]
  [omega_m3] -> Divide/2
  Gain2/1 -> Gain4/1, [P_m3]
  Fcn2/1 -> Gain2/1
  Mux2/1 -> Fcn2/1
  C_p (\lambda)/1 -> Mux2/1
  Divide1/1 -> [lambda3], C_p (\lambda)/1  "lambda"
  Vw/1 -> Mux2/2, Divide1/3
  Gain/1 -> Divide1/2
  [omega_m3] -> Gain/1

== system Complete_Model/Equivalent Wind Park/300MW Eq WT10/Grid Side Control ==
  Vbus_ref : Inport
  Qg_ref : Inport  Port=2
  Vbus : Inport  Port=3
  Ig_mes : Inport  Port=4
  Vs_mes : Inport  Port=5
  Demux : Demux  Outputs=2
  Demux1 : Demux  Outputs=2
  Gain : Gain  Gain=Kqg
  Gain1 : Gain  Gain=Kpg
  Normalized for PWM : Gain  Gain=1/(Vbus/2)
  "PI " : Subsystem
  PI id : Subsystem
  PI iq : Subsystem
  Sum : Sum  Inputs=|++
  Vs_mes1 : InportShadow  Port=5
  abc->alpha/beta : Subsystem
  abc->alpha/beta1 : Subsystem
  alpha/beta->abc : Subsystem
  alpha/beta->d/q : Subsystem
  alpha/beta->d/q1 : Subsystem
  angle2 : Subsystem
  cancellation of coupling terms : Subsystem
  d/q->alpha/beta : Subsystem
  Vabc_ref : Outport
  tag scope: Qg_ref3=global, Vbus_ref3=global, idg3=global, idg_ref3=global, iqg3=global, iqg_ref3=global, vdg3=global, vqg3=global
  wires:
  Vs_mes1/1 -> Sum/2
  angle2/1(theta_g) -> [theta_grid3]
  [theta_grid3] -> alpha/beta->d/q1/2(theta)
  [theta_grid3] -> d/q->alpha/beta/2(theta)
  [theta_grid3] -> alpha/beta->d/q/2(theta)
  Demux/2 -> PI iq/2(meas), [iqg3]
  Demux/1 -> PI id/2(meas), [idg3]
  Vbus/1 -> "PI "/2(meas)
  Demux1/1 -> [vdg3]
  Demux1/2 -> [vqg3]
  alpha/beta->d/q1/1(d/q) -> Demux1/1
  abc->alpha/beta1/1(alpha/beta) -> alpha/beta->d/q1/1(D/Q)
  [Vg3] -> abc->alpha/beta1/1(abc)
  cancellation of coupling terms/1(out) -> d/q->alpha/beta/1(d/q)
  Sum/1 -> Normalized for PWM/1
  alpha/beta->abc/1(abc) -> Sum/1
  Qg_ref/1 -> Gain/1, [Qg_ref3]
  Gain1/1 -> PI id/1(ref), [idg_ref3]  "idg"
  "PI "/1(out) -> Gain1/1  "Pg_ref"
  Gain/1 -> [iqg_ref3], PI iq/1(ref)  "iqg"
  Vbus_ref/1 -> "PI "/1(ref), [Vbus_ref3]
  alpha/beta->d/q/1(d/q) -> Demux/1
  PI iq/1(out) -> cancellation of coupling terms/2(Vq*)
  PI id/1(out) -> cancellation of coupling terms/1(Vd*)
  Vs_mes/1 -> angle2/1(Vs_mes)
  Normalized for PWM/1 -> Vabc_ref/1
  abc->alpha/beta/1(alpha/beta) -> alpha/beta->d/q/1(D/Q)
  Ig_mes/1 -> abc->alpha/beta/1(abc)
  d/q->alpha/beta/1(D/Q) -> alpha/beta->abc/1(D/Q)

== system Complete_Model/Equivalent Wind Park/300MW Eq WT10/Power Calculations ==
  Demux{,1..3} (4 alike) : Demux  Outputs=2
  Divide : Product  Inputs=**
  Gain : Gain  Gain=3/2
  Gain1 : Gain  Gain=-1
  Gain2 : Gain  Gain=-1
  Power (3ph, Instantaneous){1..4} (4 alike) : spsPower3phInstantaneousLib/Power (3ph, Instantaneous)
  Scope{1..8,10..13} (12 alike) : Scope
  Scope9 : Scope  NumInputPorts=2
  "Second-Order " : spsSecondOrderFilterLib/Second-Order Filter  FilterType=Lowpass  Fo=17  Zeta=0.7  Ts=7.4074e-05  Initialize=off  Vac_Init="[0,  0,  60]"  Vdc_Init=0  PlotResponse=off  FreqRange=[0, 500, 1]
      settings: AttributesFormatString=f0=%<Fo>
  Second-Order 1 : spsSecondOrderFilterLib/Second-Order Filter  FilterType=Lowpass  Fo=17  Zeta=0.7  Ts=7.4074e-05  Initialize=off  Vac_Init="[0,  0,  60]"  Vdc_Init=0  PlotResponse=off  FreqRange=[0, 500, 1]
      settings: AttributesFormatString=f0=%<Fo>
  Second-Order Low-Pass Filter {1..7} (7 alike) : spsSecondOrderFilterLib/Second-Order Filter  FilterType=Lowpass  Fo=17  Zeta=0.7  Ts=7.4074e-05  Initialize=off  Vac_Init="[0,  0,  60]"  Vdc_Init=0  PlotResponse=off  FreqRange=[0, 500, 1]
      settings: AttributesFormatString=f0=%<Fo>
  Subsystem : Subsystem
  Subsystem1 : Subsystem
  Subsystem2 : Subsystem
  Subsystem3 : Subsystem
  To Workspace1 : ToWorkspace  VariableName=Iout_d3  SaveFormat=Timeseries  SampleTime=-1
  To Workspace2 : ToWorkspace  VariableName=Ig_d3  SaveFormat=Timeseries  SampleTime=-1
  To Workspace21 : ToWorkspace  VariableName=Vout_d3  SaveFormat=Timeseries  SampleTime=-1
  To Workspace3 : ToWorkspace  VariableName=Vg_d3  SaveFormat=Timeseries  SampleTime=-1
  ToFile_Ig_d3 : ToFile  Filename=Ig_d3.mat  SaveFormat=Timeseries
  ToFile_Iout_d3 : ToFile  Filename=Iout_d3.mat  SaveFormat=Timeseries
  ToFile_Vg_d3 : ToFile  Filename=Vg_d3.mat  SaveFormat=Timeseries
  ToFile_Vout_d3 : ToFile  Filename=Vout_d3.mat  SaveFormat=Timeseries
  alpha/beta->d/q : Subsystem
  alpha/beta->d/q1 : Subsystem
  alpha/beta->d/q2 : Subsystem
  alpha/beta->d/q3 : Subsystem
  angle1 : Subsystem
  angle2 : Subsystem
  tag scope: Pg_mes3=global, Pout3=global, Pr_mes_machine3=global, Ps_mes_machine3=global, Qg_mes3=global, Qout3=global, Qr_mes_machine3=global, Qs_mes_machine3=global
  wires:
  [Vg3] -> angle1/1(Vs_mes), Subsystem2/1(abc)
  Subsystem2/1(alpha/beta) -> alpha/beta->d/q2/1(D/Q)
  alpha/beta->d/q2/1(d/q) -> Demux2/1
  Demux2/1 -> To Workspace3/1, Scope10/1, ToFile_Vg_d3/1  "Vg_d3"
  Demux2/2 -> Scope11/1
  Demux3/2 -> Scope13/1
  Demux3/1 -> Scope12/1, To Workspace2/1, ToFile_Ig_d3/1  "Ig_d3"
  alpha/beta->d/q3/1(d/q) -> Demux3/1
  Subsystem3/1(alpha/beta) -> alpha/beta->d/q3/1(D/Q)
  angle1/1(theta_g) -> alpha/beta->d/q3/2(theta), alpha/beta->d/q2/2(theta)
  [Ig3] -> Subsystem3/1(abc)
  Gain2/1 -> Second-Order 1/1
  Gain/1 -> Gain2/1
  Gain1/1 -> Scope9/2
  Second-Order 1/1 -> Scope9/1  "Pout"
  Divide/1 -> Gain/1
  [Iout3] -> Subsystem1/1(abc)
  angle2/1(theta_g) -> alpha/beta->d/q/2(theta), alpha/beta->d/q1/2(theta)
  Subsystem1/1(alpha/beta) -> alpha/beta->d/q1/1(D/Q)
  alpha/beta->d/q1/1(d/q) -> Demux1/1
  Demux1/1 -> To Workspace1/1, Scope7/1, Divide/2, ToFile_Iout_d3/1  "Iout_d3"
  Demux1/2 -> Scope8/1
  Demux/2 -> Scope6/1
  Demux/1 -> Scope2/1, To Workspace21/1, Divide/1, ToFile_Vout_d3/1  "Vout_d3"
  alpha/beta->d/q/1(d/q) -> Demux/1
  Subsystem/1(alpha/beta) -> alpha/beta->d/q/1(D/Q)
  [Vout3] -> Subsystem/1(abc), angle2/1(Vs_mes)
  [Vr_mes3] -> "Power (3ph, Instantaneous)4"/1
  "Power (3ph, Instantaneous)1"/1 -> Second-Order Low-Pass Filter 7/1  "Pr"
  "Power (3ph, Instantaneous)4"/1 -> Second-Order Low-Pass Filter 5/1  "Pr"
  [Vout3] -> "Power (3ph, Instantaneous)2"/1
  "Power (3ph, Instantaneous)3"/2 -> Second-Order Low-Pass Filter 2/1
  [Vg3] -> "Power (3ph, Instantaneous)1"/1
  Second-Order Low-Pass Filter 2/1 -> [Qs_mes_machine3]  "Q_stator"
  [Vs_mes_machine3] -> "Power (3ph, Instantaneous)3"/1
  "Power (3ph, Instantaneous)2"/1 -> "Second-Order "/1
  Second-Order Low-Pass Filter 5/1 -> [Pr_mes_machine3]  "P_rotor"
  [Is_mes_machine3] -> "Power (3ph, Instantaneous)3"/2, Scope4/1
  Second-Order Low-Pass Filter 7/1 -> [Pg_mes3]  "P_rotor"
  [Ir_mes3] -> "Power (3ph, Instantaneous)4"/2, Scope5/1
  "Second-Order "/1 -> [Pout3], Gain1/1  "Pout"
  Second-Order Low-Pass Filter 6/1 -> [Qg_mes3]  "Q_rotor"
  Second-Order Low-Pass Filter 4/1 -> [Qr_mes_machine3]  "Q_rotor"
  "Power (3ph, Instantaneous)3"/1 -> Second-Order Low-Pass Filter 3/1  "Ps"
  [Ig3] -> Scope1/1, "Power (3ph, Instantaneous)1"/2
  "Power (3ph, Instantaneous)4"/2 -> Second-Order Low-Pass Filter 4/1
  [Iout3] -> "Power (3ph, Instantaneous)2"/2, Scope3/1
  "Power (3ph, Instantaneous)1"/2 -> Second-Order Low-Pass Filter 6/1
  Second-Order Low-Pass Filter 3/1 -> [Ps_mes_machine3]  "P_stator"
  Second-Order Low-Pass Filter 1/1 -> [Qout3]  "Qout"
  "Power (3ph, Instantaneous)2"/2 -> Second-Order Low-Pass Filter 1/1

== system Complete_Model/Equivalent Wind Park/300MW Eq WT10/Rotor Side Control ==
  note: Stator referred
  idr_ref : Inport
  Ir_mes : Inport  Port=2
  Vs_mes : Inport  Port=3
  theta_m_mes : Inport  Port=4
  speed_mes : Inport  Port=5
  D/Q->abc : Subsystem
  D/Q->d/q : Subsystem
  Demux : Demux  Outputs=2
  Gain : Gain  Gain=1/(-1.5*p*(Lm/Ls)*Fs)
  Gain1 : Gain  Gain=1/u
  Gain2 : Gain  Gain=1/u
  MPPT : Subsystem
  Normalized for PWM : Gain  Gain=1/(Vbus/2)
  PI id : Subsystem
  PI iq : Subsystem
  abc->alpha/beta : Subsystem
  angle : Subsystem
  cancellation of coupling terms : Subsystem
  d/q->D/Q : Subsystem
  Vabc_ref : Outport
  tag scope: Tr_ref3=global, idr3=global, idr_ref3=global, iqr3=global, iqr_ref3=global, vrabc_ref3=global
  wires:
  MPPT/1(Tm,opt) -> Gain/1, [Tr_ref3]
  d/q->D/Q/1(D/Q) -> D/Q->abc/1(D/Q)
  abc->alpha/beta/1(alpha/beta) -> D/Q->d/q/1(D/Q)
  Gain2/1 -> [vrabc_ref3], Normalized for PWM/1  "Rotor referred"
  D/Q->abc/1(abc) -> Gain2/1
  Normalized for PWM/1 -> Vabc_ref/1
  Gain1/1 -> abc->alpha/beta/1(abc)
  idr_ref/1 -> PI id/1(ref), [idr_ref3]
  Gain/1 -> PI iq/1(ref), [iqr_ref3]
  Demux/2 -> PI iq/2(meas), [iqr3]
  Demux/1 -> [idr3], PI id/2(meas)
  D/Q->d/q/1(d/q) -> Demux/1  "i_dq = rotor ref frame"
  speed_mes/1 -> MPPT/1(omega_m), cancellation of coupling terms/3(omega_m)
  cancellation of coupling terms/1(out) -> d/q->D/Q/1(d/q)  "Vdr_ref,Vqr_ref"
  PI iq/1(out) -> cancellation of coupling terms/2(Vq*)
  PI id/1(out) -> cancellation of coupling terms/1(Vd*)
  angle/1(theta_r) -> D/Q->d/q/2(theta), d/q->D/Q/2(theta)
  Vs_mes/1 -> angle/1(Vs_mes)
  theta_m_mes/1 -> angle/2(theta_m)
  Ir_mes/1 -> Gain1/1

== system Complete_Model/Equivalent Wind Park/300MW Eq WT10/Subsystem ==
  In1 : Inport
  Bus Selector : BusSelector  OutputSignals=Mechanical.Rotor angle thetam (rad),Mechanical.Rotor speed (wm),Mechanical.Electromagnetic torque Te (N*m)
  Second-Order Low-Pass Filter 3 : spsSecondOrderFilterLib/Second-Order Filter  FilterType=Lowpass  Fo=17  Zeta=0.7  Ts=7.4074e-05  Initialize=off  Vac_Init="[0,  0,  60]"  Vdc_Init=0  PlotResponse=off  FreqRange=[0, 500, 1]
      settings: AttributesFormatString=f0=%<Fo>
  tag scope: Tr3=global, omega_m3=global, theta3=global
  wires:
  Second-Order Low-Pass Filter 3/1 -> [omega_m3]
  Bus Selector/3 -> [Tr3]  "<Electromagnetic torque Te (N*m)>"
  Bus Selector/1 -> [theta3]  "<Rotor angle thetam (rad)>"
  In1/1 -> Bus Selector/1
  Bus Selector/2 -> Second-Order Low-Pass Filter 3/1  "<Rotor speed (wm)>"
